simulink library "Garbage"  (R2023a)
settings: Solver=ode45  StartTime=0.0  StopTime=10.0  RelTol=1e-3  EnableMultiTasking=on
undefined symbols (not defined in the file or in its folder): c, ending_column_index, ending_row_index, framebuffer_16x1, framebuffer_2x2, framebuffer_4x4, framebuffer_8x8, HEIGHT, hot, POS_X, POS_Y, starting_column_index, starting_row_index, WIDTH
libraries: Memory_Blocks_Lib x459, simulink x235, simulink_extras x132, dspmtrx3 x116, Bit_Multiplexers_Lib x67, Bit_Demultiplexers_Lib x49, Byte_Multiplexers_Lib x40, Miscellaneous x38, Computer_Library x17, Decoders_Lib x14, commutil2 x12, Arithmetic_Operations_Lib x8
(part 25 of 26)

== system Garbage/Text_Display_1x16/Character_Table/Characters(0..F)/Char_D ==
  Byte_01 : Constant  Value=c.char(2, c.D)  OutDataTypeStr=uint8
  Byte_02 : Constant  Value=c.char(3, c.D)  OutDataTypeStr=uint8
  Byte_03 : Constant  Value=c.char(4, c.D)  OutDataTypeStr=uint8
  Byte_04 : Constant  Value=c.char(5, c.D)  OutDataTypeStr=uint8
  Byte_05 : Constant  Value=c.char(6, c.D)  OutDataTypeStr=uint8
  Byte_06 : Constant  Value=c.char(7, c.D)  OutDataTypeStr=uint8
  Byte_07 : Constant  Value=c.char(8, c.D)  OutDataTypeStr=uint8
  Byte_1 : Constant  Value=c.char(1, c.D)  OutDataTypeStr=uint8
  Row_7(1B) : Outport
  Row_6(1B) : Outport  Port=2
  Row_5(1B) : Outport  Port=3
  Row_4(1B) : Outport  Port=4
  Row_3(1B) : Outport  Port=5
  Row_2(1B) : Outport  Port=6
  Row_1(1B) : Outport  Port=7
  Row_0(1B) : Outport  Port=8
  wires:
  Byte_02/1 -> Row_5(1B)/1
  Byte_1/1 -> Row_7(1B)/1
  Byte_06/1 -> Row_1(1B)/1
  Byte_03/1 -> Row_4(1B)/1
  Byte_07/1 -> Row_0(1B)/1
  Byte_01/1 -> Row_6(1B)/1
  Byte_04/1 -> Row_3(1B)/1
  Byte_05/1 -> Row_2(1B)/1

== system Garbage/Text_Display_1x16/Character_Table/Characters(0..F)/Char_E ==
  Byte_01 : Constant  Value=c.char(2, c.E)  OutDataTypeStr=uint8
  Byte_02 : Constant  Value=c.char(3, c.E)  OutDataTypeStr=uint8
  Byte_03 : Constant  Value=c.char(4, c.E)  OutDataTypeStr=uint8
  Byte_04 : Constant  Value=c.char(5, c.E)  OutDataTypeStr=uint8
  Byte_05 : Constant  Value=c.char(6, c.E)  OutDataTypeStr=uint8
  Byte_06 : Constant  Value=c.char(7, c.E)  OutDataTypeStr=uint8
  Byte_07 : Constant  Value=c.char(8, c.E)  OutDataTypeStr=uint8
  Byte_1 : Constant  Value=c.char(1, c.E)  OutDataTypeStr=uint8
  Row_7(1B) : Outport
  Row_6(1B) : Outport  Port=2
  Row_5(1B) : Outport  Port=3
  Row_4(1B) : Outport  Port=4
  Row_3(1B) : Outport  Port=5
  Row_2(1B) : Outport  Port=6
  Row_1(1B) : Outport  Port=7
  Row_0(1B) : Outport  Port=8
  wires:
  Byte_02/1 -> Row_5(1B)/1
  Byte_1/1 -> Row_7(1B)/1
  Byte_06/1 -> Row_1(1B)/1
  Byte_03/1 -> Row_4(1B)/1
  Byte_07/1 -> Row_0(1B)/1
  Byte_01/1 -> Row_6(1B)/1
  Byte_04/1 -> Row_3(1B)/1
  Byte_05/1 -> Row_2(1B)/1

== system Garbage/Text_Display_1x16/Character_Table/Characters(0..F)/Char_F ==
  Byte_01 : Constant  Value=c.char(2, c.F)  OutDataTypeStr=uint8
  Byte_02 : Constant  Value=c.char(3, c.F)  OutDataTypeStr=uint8
  Byte_03 : Constant  Value=c.char(4, c.F)  OutDataTypeStr=uint8
  Byte_04 : Constant  Value=c.char(5, c.F)  OutDataTypeStr=uint8
  Byte_05 : Constant  Value=c.char(6, c.F)  OutDataTypeStr=uint8
  Byte_06 : Constant  Value=c.char(7, c.F)  OutDataTypeStr=uint8
  Byte_07 : Constant  Value=c.char(8, c.F)  OutDataTypeStr=uint8
  Byte_1 : Constant  Value=c.char(1, c.F)  OutDataTypeStr=uint8
  Row_7(1B) : Outport
  Row_6(1B) : Outport  Port=2
  Row_5(1B) : Outport  Port=3
  Row_4(1B) : Outport  Port=4
  Row_3(1B) : Outport  Port=5
  Row_2(1B) : Outport  Port=6
  Row_1(1B) : Outport  Port=7
  Row_0(1B) : Outport  Port=8
  wires:
  Byte_02/1 -> Row_5(1B)/1
  Byte_1/1 -> Row_7(1B)/1
  Byte_06/1 -> Row_1(1B)/1
  Byte_03/1 -> Row_4(1B)/1
  Byte_07/1 -> Row_0(1B)/1
  Byte_01/1 -> Row_6(1B)/1
  Byte_04/1 -> Row_3(1B)/1
  Byte_05/1 -> Row_2(1B)/1

== system Garbage/Text_Display_1x16/Character_Table/Characters(ARROW_DOWN..RECTANGLE)/Char_ARROW_DOWN ==
  Byte_01 : Constant  Value=c.char(2, c.ARROW_DOWN)  OutDataTypeStr=uint8
  Byte_02 : Constant  Value=c.char(3, c.ARROW_DOWN)  OutDataTypeStr=uint8
  Byte_03 : Constant  Value=c.char(4, c.ARROW_DOWN)  OutDataTypeStr=uint8
  Byte_04 : Constant  Value=c.char(5, c.ARROW_DOWN)  OutDataTypeStr=uint8
  Byte_05 : Constant  Value=c.char(6, c.ARROW_DOWN)  OutDataTypeStr=uint8
  Byte_06 : Constant  Value=c.char(7, c.ARROW_DOWN)  OutDataTypeStr=uint8
  Byte_07 : Constant  Value=c.char(8, c.ARROW_DOWN)  OutDataTypeStr=uint8
  Byte_1 : Constant  Value=c.char(1, c.ARROW_DOWN)  OutDataTypeStr=uint8
  Row_7(1B) : Outport
  Row_6(1B) : Outport  Port=2
  Row_5(1B) : Outport  Port=3
  Row_4(1B) : Outport  Port=4
  Row_3(1B) : Outport  Port=5
  Row_2(1B) : Outport  Port=6
  Row_1(1B) : Outport  Port=7
  Row_0(1B) : Outport  Port=8
  wires:
  Byte_02/1 -> Row_5(1B)/1
  Byte_1/1 -> Row_7(1B)/1
  Byte_06/1 -> Row_1(1B)/1
  Byte_03/1 -> Row_4(1B)/1
  Byte_07/1 -> Row_0(1B)/1
  Byte_01/1 -> Row_6(1B)/1
  Byte_04/1 -> Row_3(1B)/1
  Byte_05/1 -> Row_2(1B)/1

== system Garbage/Text_Display_1x16/Character_Table/Characters(ARROW_DOWN..RECTANGLE)/Char_ARROW_LEFT ==
  Byte_01 : Constant  Value=c.char(2, c.ARROW_LEFT)  OutDataTypeStr=uint8
  Byte_02 : Constant  Value=c.char(3, c.ARROW_LEFT)  OutDataTypeStr=uint8
  Byte_03 : Constant  Value=c.char(4, c.ARROW_LEFT)  OutDataTypeStr=uint8
  Byte_04 : Constant  Value=c.char(5, c.ARROW_LEFT)  OutDataTypeStr=uint8
  Byte_05 : Constant  Value=c.char(6, c.ARROW_LEFT)  OutDataTypeStr=uint8
  Byte_06 : Constant  Value=c.char(7, c.ARROW_LEFT)  OutDataTypeStr=uint8
  Byte_07 : Constant  Value=c.char(8, c.ARROW_LEFT)  OutDataTypeStr=uint8
  Byte_1 : Constant  Value=c.char(1, c.ARROW_LEFT)  OutDataTypeStr=uint8
  Row_7(1B) : Outport
  Row_6(1B) : Outport  Port=2
  Row_5(1B) : Outport  Port=3
  Row_4(1B) : Outport  Port=4
  Row_3(1B) : Outport  Port=5
  Row_2(1B) : Outport  Port=6
  Row_1(1B) : Outport  Port=7
  Row_0(1B) : Outport  Port=8
  wires:
  Byte_02/1 -> Row_5(1B)/1
  Byte_1/1 -> Row_7(1B)/1
  Byte_06/1 -> Row_1(1B)/1
  Byte_03/1 -> Row_4(1B)/1
  Byte_07/1 -> Row_0(1B)/1
  Byte_01/1 -> Row_6(1B)/1
  Byte_04/1 -> Row_3(1B)/1
  Byte_05/1 -> Row_2(1B)/1

== system Garbage/Text_Display_1x16/Character_Table/Characters(ARROW_DOWN..RECTANGLE)/Char_ARROW_RIGHT ==
  Byte_01 : Constant  Value=c.char(2, c.ARROW_RIGHT)  OutDataTypeStr=uint8
  Byte_02 : Constant  Value=c.char(3, c.ARROW_RIGHT)  OutDataTypeStr=uint8
  Byte_03 : Constant  Value=c.char(4, c.ARROW_RIGHT)  OutDataTypeStr=uint8
  Byte_04 : Constant  Value=c.char(5, c.ARROW_RIGHT)  OutDataTypeStr=uint8
  Byte_05 : Constant  Value=c.char(6, c.ARROW_RIGHT)  OutDataTypeStr=uint8
  Byte_06 : Constant  Value=c.char(7, c.ARROW_RIGHT)  OutDataTypeStr=uint8
  Byte_07 : Constant  Value=c.char(8, c.ARROW_RIGHT)  OutDataTypeStr=uint8
  Byte_1 : Constant  Value=c.char(1, c.ARROW_RIGHT)  OutDataTypeStr=uint8
  Row_7(1B) : Outport
  Row_6(1B) : Outport  Port=2
  Row_5(1B) : Outport  Port=3
  Row_4(1B) : Outport  Port=4
  Row_3(1B) : Outport  Port=5
  Row_2(1B) : Outport  Port=6
  Row_1(1B) : Outport  Port=7
  Row_0(1B) : Outport  Port=8
  wires:
  Byte_02/1 -> Row_5(1B)/1
  Byte_1/1 -> Row_7(1B)/1
  Byte_06/1 -> Row_1(1B)/1
  Byte_03/1 -> Row_4(1B)/1
  Byte_07/1 -> Row_0(1B)/1
  Byte_01/1 -> Row_6(1B)/1
  Byte_04/1 -> Row_3(1B)/1
  Byte_05/1 -> Row_2(1B)/1

== system Garbage/Text_Display_1x16/Character_Table/Characters(ARROW_DOWN..RECTANGLE)/Char_COMMA ==
  Byte_01 : Constant  Value=c.char(2, c.COMMA)  OutDataTypeStr=uint8
  Byte_02 : Constant  Value=c.char(3, c.COMMA)  OutDataTypeStr=uint8
  Byte_03 : Constant  Value=c.char(4, c.COMMA)  OutDataTypeStr=uint8
  Byte_04 : Constant  Value=c.char(5, c.COMMA)  OutDataTypeStr=uint8
  Byte_05 : Constant  Value=c.char(6, c.COMMA)  OutDataTypeStr=uint8
  Byte_06 : Constant  Value=c.char(7, c.COMMA)  OutDataTypeStr=uint8
  Byte_07 : Constant  Value=c.char(8, c.COMMA)  OutDataTypeStr=uint8
  Byte_1 : Constant  Value=c.char(1, c.COMMA)  OutDataTypeStr=uint8
  Row_7(1B) : Outport
  Row_6(1B) : Outport  Port=2
  Row_5(1B) : Outport  Port=3
  Row_4(1B) : Outport  Port=4
  Row_3(1B) : Outport  Port=5
  Row_2(1B) : Outport  Port=6
  Row_1(1B) : Outport  Port=7
  Row_0(1B) : Outport  Port=8
  wires:
  Byte_02/1 -> Row_5(1B)/1
  Byte_1/1 -> Row_7(1B)/1
  Byte_06/1 -> Row_1(1B)/1
  Byte_03/1 -> Row_4(1B)/1
  Byte_07/1 -> Row_0(1B)/1
  Byte_01/1 -> Row_6(1B)/1
  Byte_04/1 -> Row_3(1B)/1
  Byte_05/1 -> Row_2(1B)/1

== system Garbage/Text_Display_1x16/Character_Table/Characters(ARROW_DOWN..RECTANGLE)/Char_CURSOR ==
  Byte_01 : Constant  Value=c.char(2, c.CURSOR)  OutDataTypeStr=uint8
  Byte_02 : Constant  Value=c.char(3, c.CURSOR)  OutDataTypeStr=uint8
  Byte_03 : Constant  Value=c.char(4, c.CURSOR)  OutDataTypeStr=uint8
  Byte_04 : Constant  Value=c.char(5, c.CURSOR)  OutDataTypeStr=uint8
  Byte_05 : Constant  Value=c.char(6, c.CURSOR)  OutDataTypeStr=uint8
  Byte_06 : Constant  Value=c.char(7, c.CURSOR)  OutDataTypeStr=uint8
  Byte_07 : Constant  Value=c.char(8, c.CURSOR)  OutDataTypeStr=uint8
  Byte_1 : Constant  Value=c.char(1, c.CURSOR)  OutDataTypeStr=uint8
  Row_7(1B) : Outport
  Row_6(1B) : Outport  Port=2
  Row_5(1B) : Outport  Port=3
  Row_4(1B) : Outport  Port=4
  Row_3(1B) : Outport  Port=5
  Row_2(1B) : Outport  Port=6
  Row_1(1B) : Outport  Port=7
  Row_0(1B) : Outport  Port=8
  wires:
  Byte_02/1 -> Row_5(1B)/1
  Byte_1/1 -> Row_7(1B)/1
  Byte_06/1 -> Row_1(1B)/1
  Byte_03/1 -> Row_4(1B)/1
  Byte_07/1 -> Row_0(1B)/1
  Byte_01/1 -> Row_6(1B)/1
  Byte_04/1 -> Row_3(1B)/1
  Byte_05/1 -> Row_2(1B)/1

== system Garbage/Text_Display_1x16/Character_Table/Characters(ARROW_DOWN..RECTANGLE)/Char_DOT ==
  Byte_01 : Constant  Value=c.char(2, c.DOT)  OutDataTypeStr=uint8
  Byte_02 : Constant  Value=c.char(3, c.DOT)  OutDataTypeStr=uint8
  Byte_03 : Constant  Value=c.char(4, c.DOT)  OutDataTypeStr=uint8
  Byte_04 : Constant  Value=c.char(5, c.DOT)  OutDataTypeStr=uint8
  Byte_05 : Constant  Value=c.char(6, c.DOT)  OutDataTypeStr=uint8
  Byte_06 : Constant  Value=c.char(7, c.DOT)  OutDataTypeStr=uint8
  Byte_07 : Constant  Value=c.char(8, c.DOT)  OutDataTypeStr=uint8
  Byte_1 : Constant  Value=c.char(1, c.DOT)  OutDataTypeStr=uint8
  Row_7(1B) : Outport
  Row_6(1B) : Outport  Port=2
  Row_5(1B) : Outport  Port=3
  Row_4(1B) : Outport  Port=4
  Row_3(1B) : Outport  Port=5
  Row_2(1B) : Outport  Port=6
  Row_1(1B) : Outport  Port=7
  Row_0(1B) : Outport  Port=8
  wires:
  Byte_02/1 -> Row_5(1B)/1
  Byte_1/1 -> Row_7(1B)/1
  Byte_06/1 -> Row_1(1B)/1
  Byte_03/1 -> Row_4(1B)/1
  Byte_07/1 -> Row_0(1B)/1
  Byte_01/1 -> Row_6(1B)/1
  Byte_04/1 -> Row_3(1B)/1
  Byte_05/1 -> Row_2(1B)/1

== system Garbage/Text_Display_1x16/Character_Table/Characters(ARROW_DOWN..RECTANGLE)/Char_EXCLAMATION_MARK ==
  Byte_01 : Constant  Value=c.char(2, c.EXCLAMATION_MARK)  OutDataTypeStr=uint8
  Byte_02 : Constant  Value=c.char(3, c.EXCLAMATION_MARK)  OutDataTypeStr=uint8
  Byte_03 : Constant  Value=c.char(4, c.EXCLAMATION_MARK)  OutDataTypeStr=uint8
  Byte_04 : Constant  Value=c.char(5, c.EXCLAMATION_MARK)  OutDataTypeStr=uint8
  Byte_05 : Constant  Value=c.char(6, c.EXCLAMATION_MARK)  OutDataTypeStr=uint8
  Byte_06 : Constant  Value=c.char(7, c.EXCLAMATION_MARK)  OutDataTypeStr=uint8
  Byte_07 : Constant  Value=c.char(8, c.EXCLAMATION_MARK)  OutDataTypeStr=uint8
  Byte_1 : Constant  Value=c.char(1, c.EXCLAMATION_MARK)  OutDataTypeStr=uint8
  Row_7(1B) : Outport
  Row_6(1B) : Outport  Port=2
  Row_5(1B) : Outport  Port=3
  Row_4(1B) : Outport  Port=4
  Row_3(1B) : Outport  Port=5
  Row_2(1B) : Outport  Port=6
  Row_1(1B) : Outport  Port=7
  Row_0(1B) : Outport  Port=8
  wires:
  Byte_02/1 -> Row_5(1B)/1
  Byte_1/1 -> Row_7(1B)/1
  Byte_06/1 -> Row_1(1B)/1
  Byte_03/1 -> Row_4(1B)/1
  Byte_07/1 -> Row_0(1B)/1
  Byte_01/1 -> Row_6(1B)/1
  Byte_04/1 -> Row_3(1B)/1
  Byte_05/1 -> Row_2(1B)/1

== system Garbage/Text_Display_1x16/Character_Table/Characters(ARROW_DOWN..RECTANGLE)/Char_LEFT_PARENTHESES ==
  Byte_01 : Constant  Value=c.char(2, c.LEFT_PARENTHESES)  OutDataTypeStr=uint8
  Byte_02 : Constant  Value=c.char(3, c.LEFT_PARENTHESES)  OutDataTypeStr=uint8
  Byte_03 : Constant  Value=c.char(4, c.LEFT_PARENTHESES)  OutDataTypeStr=uint8
  Byte_04 : Constant  Value=c.char(5, c.LEFT_PARENTHESES)  OutDataTypeStr=uint8
  Byte_05 : Constant  Value=c.char(6, c.LEFT_PARENTHESES)  OutDataTypeStr=uint8
  Byte_06 : Constant  Value=c.char(7, c.LEFT_PARENTHESES)  OutDataTypeStr=uint8
  Byte_07 : Constant  Value=c.char(8, c.LEFT_PARENTHESES)  OutDataTypeStr=uint8
  Byte_1 : Constant  Value=c.char(1, c.LEFT_PARENTHESES)  OutDataTypeStr=uint8
  Row_7(1B) : Outport
  Row_6(1B) : Outport  Port=2
  Row_5(1B) : Outport  Port=3
  Row_4(1B) : Outport  Port=4
  Row_3(1B) : Outport  Port=5
  Row_2(1B) : Outport  Port=6
  Row_1(1B) : Outport  Port=7
  Row_0(1B) : Outport  Port=8
  wires:
  Byte_02/1 -> Row_5(1B)/1
  Byte_1/1 -> Row_7(1B)/1
  Byte_06/1 -> Row_1(1B)/1
  Byte_03/1 -> Row_4(1B)/1
  Byte_07/1 -> Row_0(1B)/1
  Byte_01/1 -> Row_6(1B)/1
  Byte_04/1 -> Row_3(1B)/1
  Byte_05/1 -> Row_2(1B)/1

== system Garbage/Text_Display_1x16/Character_Table/Characters(ARROW_DOWN..RECTANGLE)/Char_PERCENT ==
  Byte_01 : Constant  Value=c.char(2, c.PERCENT)  OutDataTypeStr=uint8
  Byte_02 : Constant  Value=c.char(3, c.PERCENT)  OutDataTypeStr=uint8
  Byte_03 : Constant  Value=c.char(4, c.PERCENT)  OutDataTypeStr=uint8
  Byte_04 : Constant  Value=c.char(5, c.PERCENT)  OutDataTypeStr=uint8
  Byte_05 : Constant  Value=c.char(6, c.PERCENT)  OutDataTypeStr=uint8
  Byte_06 : Constant  Value=c.char(7, c.PERCENT)  OutDataTypeStr=uint8
  Byte_07 : Constant  Value=c.char(8, c.PERCENT)  OutDataTypeStr=uint8
  Byte_1 : Constant  Value=c.char(1, c.PERCENT)  OutDataTypeStr=uint8
  Row_7(1B) : Outport
  Row_6(1B) : Outport  Port=2
  Row_5(1B) : Outport  Port=3
  Row_4(1B) : Outport  Port=4
  Row_3(1B) : Outport  Port=5
  Row_2(1B) : Outport  Port=6
  Row_1(1B) : Outport  Port=7
  Row_0(1B) : Outport  Port=8
  wires:
  Byte_02/1 -> Row_5(1B)/1
  Byte_1/1 -> Row_7(1B)/1
  Byte_06/1 -> Row_1(1B)/1
  Byte_03/1 -> Row_4(1B)/1
  Byte_07/1 -> Row_0(1B)/1
  Byte_01/1 -> Row_6(1B)/1
  Byte_04/1 -> Row_3(1B)/1
  Byte_05/1 -> Row_2(1B)/1

== system Garbage/Text_Display_1x16/Character_Table/Characters(ARROW_DOWN..RECTANGLE)/Char_QUESTION_MARK ==
  Byte_01 : Constant  Value=c.char(2, c.QUESTION_MARK)  OutDataTypeStr=uint8
  Byte_02 : Constant  Value=c.char(3, c.QUESTION_MARK)  OutDataTypeStr=uint8
  Byte_03 : Constant  Value=c.char(4, c.QUESTION_MARK)  OutDataTypeStr=uint8
  Byte_04 : Constant  Value=c.char(5, c.QUESTION_MARK)  OutDataTypeStr=uint8
  Byte_05 : Constant  Value=c.char(6, c.QUESTION_MARK)  OutDataTypeStr=uint8
  Byte_06 : Constant  Value=c.char(7, c.QUESTION_MARK)  OutDataTypeStr=uint8
  Byte_07 : Constant  Value=c.char(8, c.QUESTION_MARK)  OutDataTypeStr=uint8
  Byte_1 : Constant  Value=c.char(1, c.QUESTION_MARK)  OutDataTypeStr=uint8
  Row_7(1B) : Outport
  Row_6(1B) : Outport  Port=2
  Row_5(1B) : Outport  Port=3
  Row_4(1B) : Outport  Port=4
  Row_3(1B) : Outport  Port=5
  Row_2(1B) : Outport  Port=6
  Row_1(1B) : Outport  Port=7
  Row_0(1B) : Outport  Port=8
  wires:
  Byte_02/1 -> Row_5(1B)/1
  Byte_1/1 -> Row_7(1B)/1
  Byte_06/1 -> Row_1(1B)/1
  Byte_03/1 -> Row_4(1B)/1
  Byte_07/1 -> Row_0(1B)/1
  Byte_01/1 -> Row_6(1B)/1
  Byte_04/1 -> Row_3(1B)/1
  Byte_05/1 -> Row_2(1B)/1

== system Garbage/Text_Display_1x16/Character_Table/Characters(ARROW_DOWN..RECTANGLE)/Char_QUOTE ==
  Byte_01 : Constant  Value=c.char(2, c.QUOTE)  OutDataTypeStr=uint8
  Byte_02 : Constant  Value=c.char(3, c.QUOTE)  OutDataTypeStr=uint8
  Byte_03 : Constant  Value=c.char(4, c.QUOTE)  OutDataTypeStr=uint8
  Byte_04 : Constant  Value=c.char(5, c.QUOTE)  OutDataTypeStr=uint8
  Byte_05 : Constant  Value=c.char(6, c.QUOTE)  OutDataTypeStr=uint8
  Byte_06 : Constant  Value=c.char(7, c.QUOTE)  OutDataTypeStr=uint8
  Byte_07 : Constant  Value=c.char(8, c.QUOTE)  OutDataTypeStr=uint8
  Byte_1 : Constant  Value=c.char(1, c.QUOTE)  OutDataTypeStr=uint8
  Row_7(1B) : Outport
  Row_6(1B) : Outport  Port=2
  Row_5(1B) : Outport  Port=3
  Row_4(1B) : Outport  Port=4
  Row_3(1B) : Outport  Port=5
  Row_2(1B) : Outport  Port=6
  Row_1(1B) : Outport  Port=7
  Row_0(1B) : Outport  Port=8
  wires:
  Byte_02/1 -> Row_5(1B)/1
  Byte_1/1 -> Row_7(1B)/1
  Byte_06/1 -> Row_1(1B)/1
  Byte_03/1 -> Row_4(1B)/1
  Byte_07/1 -> Row_0(1B)/1
  Byte_01/1 -> Row_6(1B)/1
  Byte_04/1 -> Row_3(1B)/1
  Byte_05/1 -> Row_2(1B)/1

== system Garbage/Text_Display_1x16/Character_Table/Characters(ARROW_DOWN..RECTANGLE)/Char_RECTANGLE ==
  Byte_01 : Constant  Value=c.char(2, c.RECTANGLE)  OutDataTypeStr=uint8
  Byte_02 : Constant  Value=c.char(3, c.RECTANGLE)  OutDataTypeStr=uint8
  Byte_03 : Constant  Value=c.char(4, c.RECTANGLE)  OutDataTypeStr=uint8
  Byte_04 : Constant  Value=c.char(5, c.RECTANGLE)  OutDataTypeStr=uint8
  Byte_05 : Constant  Value=c.char(6, c.RECTANGLE)  OutDataTypeStr=uint8
  Byte_06 : Constant  Value=c.char(7, c.RECTANGLE)  OutDataTypeStr=uint8
  Byte_07 : Constant  Value=c.char(8, c.RECTANGLE)  OutDataTypeStr=uint8
  Byte_1 : Constant  Value=c.char(1, c.RECTANGLE)  OutDataTypeStr=uint8
  Row_7(1B) : Outport
  Row_6(1B) : Outport  Port=2
  Row_5(1B) : Outport  Port=3
  Row_4(1B) : Outport  Port=4
  Row_3(1B) : Outport  Port=5
  Row_2(1B) : Outport  Port=6
  Row_1(1B) : Outport  Port=7
  Row_0(1B) : Outport  Port=8
  wires:
  Byte_02/1 -> Row_5(1B)/1
  Byte_1/1 -> Row_7(1B)/1
  Byte_06/1 -> Row_1(1B)/1
  Byte_03/1 -> Row_4(1B)/1
  Byte_07/1 -> Row_0(1B)/1
  Byte_01/1 -> Row_6(1B)/1
  Byte_04/1 -> Row_3(1B)/1
  Byte_05/1 -> Row_2(1B)/1

== system Garbage/Text_Display_1x16/Character_Table/Characters(ARROW_DOWN..RECTANGLE)/Char_RIGHT_PARENTHESES ==
  Byte_01 : Constant  Value=c.char(2, c.RIGHT_PARENTHESES)  OutDataTypeStr=uint8
  Byte_02 : Constant  Value=c.char(3, c.RIGHT_PARENTHESES)  OutDataTypeStr=uint8
  Byte_03 : Constant  Value=c.char(4, c.RIGHT_PARENTHESES)  OutDataTypeStr=uint8
  Byte_04 : Constant  Value=c.char(5, c.RIGHT_PARENTHESES)  OutDataTypeStr=uint8
  Byte_05 : Constant  Value=c.char(6, c.RIGHT_PARENTHESES)  OutDataTypeStr=uint8
  Byte_06 : Constant  Value=c.char(7, c.RIGHT_PARENTHESES)  OutDataTypeStr=uint8
  Byte_07 : Constant  Value=c.char(8, c.RIGHT_PARENTHESES)  OutDataTypeStr=uint8
  Byte_1 : Constant  Value=c.char(1, c.RIGHT_PARENTHESES)  OutDataTypeStr=uint8
  Row_7(1B) : Outport
  Row_6(1B) : Outport  Port=2
  Row_5(1B) : Outport  Port=3
  Row_4(1B) : Outport  Port=4
  Row_3(1B) : Outport  Port=5
  Row_2(1B) : Outport  Port=6
  Row_1(1B) : Outport  Port=7
  Row_0(1B) : Outport  Port=8
  wires:
  Byte_02/1 -> Row_5(1B)/1
  Byte_1/1 -> Row_7(1B)/1
  Byte_06/1 -> Row_1(1B)/1
  Byte_03/1 -> Row_4(1B)/1
  Byte_07/1 -> Row_0(1B)/1
  Byte_01/1 -> Row_6(1B)/1
  Byte_04/1 -> Row_3(1B)/1
  Byte_05/1 -> Row_2(1B)/1

== system Garbage/Text_Display_1x16/Character_Table/Characters(ARROW_DOWN..RECTANGLE)/Char_SHARP ==
  Byte_01 : Constant  Value=c.char(2, c.SHARP)  OutDataTypeStr=uint8
  Byte_02 : Constant  Value=c.char(3, c.SHARP)  OutDataTypeStr=uint8
  Byte_03 : Constant  Value=c.char(4, c.SHARP)  OutDataTypeStr=uint8
  Byte_04 : Constant  Value=c.char(5, c.SHARP)  OutDataTypeStr=uint8
  Byte_05 : Constant  Value=c.char(6, c.SHARP)  OutDataTypeStr=uint8
  Byte_06 : Constant  Value=c.char(7, c.SHARP)  OutDataTypeStr=uint8
  Byte_07 : Constant  Value=c.char(8, c.SHARP)  OutDataTypeStr=uint8
  Byte_1 : Constant  Value=c.char(1, c.SHARP)  OutDataTypeStr=uint8
  Row_7(1B) : Outport
  Row_6(1B) : Outport  Port=2
  Row_5(1B) : Outport  Port=3
  Row_4(1B) : Outport  Port=4
  Row_3(1B) : Outport  Port=5
  Row_2(1B) : Outport  Port=6
  Row_1(1B) : Outport  Port=7
  Row_0(1B) : Outport  Port=8
  wires:
  Byte_02/1 -> Row_5(1B)/1
  Byte_1/1 -> Row_7(1B)/1
  Byte_06/1 -> Row_1(1B)/1
  Byte_03/1 -> Row_4(1B)/1
  Byte_07/1 -> Row_0(1B)/1
  Byte_01/1 -> Row_6(1B)/1
  Byte_04/1 -> Row_3(1B)/1
  Byte_05/1 -> Row_2(1B)/1

== system Garbage/Text_Display_1x16/Character_Table/Characters(ARROW_DOWN..RECTANGLE)/Char_SPACE ==
  Byte_01 : Constant  Value=c.char(2, c.SPACE)  OutDataTypeStr=uint8
  Byte_02 : Constant  Value=c.char(3, c.SPACE)  OutDataTypeStr=uint8
  Byte_03 : Constant  Value=c.char(4, c.SPACE)  OutDataTypeStr=uint8
  Byte_04 : Constant  Value=c.char(5, c.SPACE)  OutDataTypeStr=uint8
  Byte_05 : Constant  Value=c.char(6, c.SPACE)  OutDataTypeStr=uint8
  Byte_06 : Constant  Value=c.char(7, c.SPACE)  OutDataTypeStr=uint8
  Byte_07 : Constant  Value=c.char(8, c.SPACE)  OutDataTypeStr=uint8
  Byte_1 : Constant  Value=c.char(1, c.SPACE)  OutDataTypeStr=uint8
  Row_7(1B) : Outport
  Row_6(1B) : Outport  Port=2
  Row_5(1B) : Outport  Port=3
  Row_4(1B) : Outport  Port=4
  Row_3(1B) : Outport  Port=5
  Row_2(1B) : Outport  Port=6
  Row_1(1B) : Outport  Port=7
  Row_0(1B) : Outport  Port=8
  wires:
  Byte_02/1 -> Row_5(1B)/1
  Byte_1/1 -> Row_7(1B)/1
  Byte_06/1 -> Row_1(1B)/1
  Byte_03/1 -> Row_4(1B)/1
  Byte_07/1 -> Row_0(1B)/1
  Byte_01/1 -> Row_6(1B)/1
  Byte_04/1 -> Row_3(1B)/1
  Byte_05/1 -> Row_2(1B)/1

== system Garbage/Text_Display_1x16/Character_Table/Characters(ARROW_DOWN..RECTANGLE)/Char_UNDERSCORE ==
  Byte_01 : Constant  Value=c.char(2, c.UNDERSCORE)  OutDataTypeStr=uint8
  Byte_02 : Constant  Value=c.char(3, c.UNDERSCORE)  OutDataTypeStr=uint8
  Byte_03 : Constant  Value=c.char(4, c.UNDERSCORE)  OutDataTypeStr=uint8
  Byte_04 : Constant  Value=c.char(5, c.UNDERSCORE)  OutDataTypeStr=uint8
  Byte_05 : Constant  Value=c.char(6, c.UNDERSCORE)  OutDataTypeStr=uint8
  Byte_06 : Constant  Value=c.char(7, c.UNDERSCORE)  OutDataTypeStr=uint8
  Byte_07 : Constant  Value=c.char(8, c.UNDERSCORE)  OutDataTypeStr=uint8
  Byte_1 : Constant  Value=c.char(1, c.UNDERSCORE)  OutDataTypeStr=uint8
  Row_7(1B) : Outport
  Row_6(1B) : Outport  Port=2
  Row_5(1B) : Outport  Port=3
  Row_4(1B) : Outport  Port=4
  Row_3(1B) : Outport  Port=5
  Row_2(1B) : Outport  Port=6
  Row_1(1B) : Outport  Port=7
  Row_0(1B) : Outport  Port=8
  wires:
  Byte_02/1 -> Row_5(1B)/1
  Byte_1/1 -> Row_7(1B)/1
  Byte_06/1 -> Row_1(1B)/1
  Byte_03/1 -> Row_4(1B)/1
  Byte_07/1 -> Row_0(1B)/1
  Byte_01/1 -> Row_6(1B)/1
  Byte_04/1 -> Row_3(1B)/1
  Byte_05/1 -> Row_2(1B)/1

== system Garbage/Text_Display_1x16/Character_Table/Characters(G..W)/Char_G ==
  Byte_01 : Constant  Value=c.char(2, c.G)  OutDataTypeStr=uint8
  Byte_02 : Constant  Value=c.char(3, c.G)  OutDataTypeStr=uint8
  Byte_03 : Constant  Value=c.char(4, c.G)  OutDataTypeStr=uint8
  Byte_04 : Constant  Value=c.char(5, c.G)  OutDataTypeStr=uint8
  Byte_05 : Constant  Value=c.char(6, c.G)  OutDataTypeStr=uint8
  Byte_06 : Constant  Value=c.char(7, c.G)  OutDataTypeStr=uint8
  Byte_07 : Constant  Value=c.char(8, c.G)  OutDataTypeStr=uint8
  Byte_1 : Constant  Value=c.char(1, c.G)  OutDataTypeStr=uint8
  Row_7(1B) : Outport
  Row_6(1B) : Outport  Port=2
  Row_5(1B) : Outport  Port=3
  Row_4(1B) : Outport  Port=4
  Row_3(1B) : Outport  Port=5
  Row_2(1B) : Outport  Port=6
  Row_1(1B) : Outport  Port=7
  Row_0(1B) : Outport  Port=8
  wires:
  Byte_02/1 -> Row_5(1B)/1
  Byte_1/1 -> Row_7(1B)/1
  Byte_06/1 -> Row_1(1B)/1
  Byte_03/1 -> Row_4(1B)/1
  Byte_07/1 -> Row_0(1B)/1
  Byte_01/1 -> Row_6(1B)/1
  Byte_04/1 -> Row_3(1B)/1
  Byte_05/1 -> Row_2(1B)/1

== system Garbage/Text_Display_1x16/Character_Table/Characters(G..W)/Char_H ==
  Byte_01 : Constant  Value=c.char(2, c.H)  OutDataTypeStr=uint8
  Byte_02 : Constant  Value=c.char(3, c.H)  OutDataTypeStr=uint8
  Byte_03 : Constant  Value=c.char(4, c.H)  OutDataTypeStr=uint8
  Byte_04 : Constant  Value=c.char(5, c.H)  OutDataTypeStr=uint8
  Byte_05 : Constant  Value=c.char(6, c.H)  OutDataTypeStr=uint8
  Byte_06 : Constant  Value=c.char(7, c.H)  OutDataTypeStr=uint8
  Byte_07 : Constant  Value=c.char(8, c.H)  OutDataTypeStr=uint8
  Byte_1 : Constant  Value=c.char(1, c.H)  OutDataTypeStr=uint8
  Row_7(1B) : Outport
  Row_6(1B) : Outport  Port=2
  Row_5(1B) : Outport  Port=3
  Row_4(1B) : Outport  Port=4
  Row_3(1B) : Outport  Port=5
  Row_2(1B) : Outport  Port=6
  Row_1(1B) : Outport  Port=7
  Row_0(1B) : Outport  Port=8
  wires:
  Byte_02/1 -> Row_5(1B)/1
  Byte_1/1 -> Row_7(1B)/1
  Byte_06/1 -> Row_1(1B)/1
  Byte_03/1 -> Row_4(1B)/1
  Byte_07/1 -> Row_0(1B)/1
  Byte_01/1 -> Row_6(1B)/1
  Byte_04/1 -> Row_3(1B)/1
  Byte_05/1 -> Row_2(1B)/1
